simulink model "ofdm_txrx_alamouti"  (Simulink 6.3)
settings: Solver=ode45  StartTime=0.0  StopTime=45000  RelTol=1e-3
InitFcn:
  | ofdm_rx_alamouti_init
StartFcn:
  | ofdm_rx_alamouti_init
values from neighbouring files (symbols this model uses):
  CPLength = 16    [ofdm_tx_alamouti_init.m]
  CRCPolynomial = hex2dec('04c11db7')    [calcTxCRC.m]    (also in ofdm_tx_alamouti_init.m)
  hi = zeros(1,length(cci))    [ofdm_rx_alamouti_init.m]
  hr = zeros(1,length(ccr))    [ofdm_rx_alamouti_init.m]
  max_CP_length = 16    [ofdm_tx_alamouti_init.m]
  max_num_baseRateSymbols = 31    [ofdm_tx_alamouti_init.m]
  max_num_subcarriers = 64    [ofdm_tx_alamouti_init.m]
  max_num_trainingSymbols = 15    [ofdm_tx_alamouti_init.m]
  max_OFDM_symbols = 2047    [ofdm_tx_alamouti_init.m]
  modConstellation_qam16 = 0.75*[1 3 -1 -3]./3    [ofdm_tx_alamouti_init.m]
  modConstellation_qam256 = 0.9375*[3 1 5 7 11 9 13 15 -3 -1 -5 -7 -11 -9 -13 -15]./15    [ofdm_tx_alamouti_init.m]
  modConstellation_qam64 = 0.875*[3 1 5 7 -3 -1 -5 -7]./7    [ofdm_tx_alamouti_init.m]
  modConstellation_qpsk = [1 -1]./sqrt(2)    [ofdm_tx_alamouti_init.m]
  numBaseRateSymbols = 2    [ofdm_tx_alamouti_init.m]
  numFullRateSymbols = numFullRateSymbols + mod(numFullRateSymbols, 2)    [ofdm_tx_alamouti_init.m]
  numSubcarriers = 64    [ofdm_tx_alamouti_init.m]
  numTrainingSymbols = 4    [ofdm_tx_alamouti_init.m]
  pkt_crossCorr_thresh = 0.7    [ofdm_rx_alamouti_init.m]
  pkt_energy_thresh = 0    [ofdm_rx_alamouti_init.m]
  pkt_numPayloadBytes = 24+4+20+20+500    (= 568)    [ofdm_tx_alamouti_init.m]
  pktTiming_count_payloadStart = pktTiming_count_lsStart + pktTiming_ls_num*pktTiming_ls_length    [ofdm_rx_init_packetTimingControl.m]
  preamble = 6*[0 shortsyms_10 longsyms_2]    [ofdm_tx_alamouti_init.m]
  preamble_I = real(preamble)    [ofdm_tx_alamouti_init.m]
  preamble_Q = imag(preamble)    [ofdm_tx_alamouti_init.m]
  RAM_init_size = 4096    [ofdm_tx_alamouti_init.m]
  rx_longCorrCountLoad = 251-16    (= 235)    [ofdm_rx_alamouti_init.m]
  rx_longCorrThresh = 3000    [ofdm_rx_alamouti_init.m]
  RxDataScrambling_Seq = [40 198 78 63 82 173 102 245 48 111 172 115 147 230 216 93 72 65 62 2 205 242 122 90 128 83 105 97 73 10 5 252]    [ofdm_tx_alamouti_init.m]
  simOnly_numSamples = length(preamble)+( (numSubcarriers+CPLength)*(numTrainingSymbols + numBaseRateSymbols + numFullRateSymbols) )    [ofdm_tx_alamouti_init.m]
  train = [train train]    [ofdm_tx_alamouti_init.m]
  TxDataScrambling_Seq = [40 198 78 63 82 173 102 245 48 111 172 115 147 230 216 93 72 65 62 2 205 242 122 90 128 83 105 97 73 10 5 252]    [ofdm_tx_alamouti_init.m]
undefined symbols (not defined in the file or in its folder): CRC_table_gen, gcbh, interpFilter_4, xlBlockLoadCallback
libraries: xbsIndex_r4 x4373, dspmlti4 x4, dspsigops x4, commchan3 x2, simulink x2, commrflib2 x2, commsink2 x2, dspsrcs4 x1
(part 14 of 39)

== system ofdm_txrx_alamouti/OFDM Rx Alamouti/FFT & Chan Est/Channel Estimation/Mult by training ==
  AR : Inport
  AI : Inport  Port=2
  BR : Inport  Port=3
  Constant1 : xbsIndex_r4/Constant  arith_type=Signed (2's comp)  const=0  n_bits=2  bin_pt=1  explicit_period=off  period=1  equ=P=C  opselect=C  inp2=PCIN>>17  opr=+  inp1=P  carry=CIN  dbl_ovrd=off  block_type=constant
      settings: block_version=VER_STRING_GOES_HERE
  Constant2 : xbsIndex_r4/Constant  arith_type=Signed (2's comp)  const=0  n_bits=2  bin_pt=1  explicit_period=off  period=1  equ=P=C  opselect=C  inp2=PCIN>>17  opr=+  inp1=P  carry=CIN  dbl_ovrd=off  block_type=constant
      settings: block_version=VER_STRING_GOES_HERE
  Mult by Training : Scope  NumInputPorts=5
      settings: TimeRange=5000  YMin=-1~-1~-1~0~0  YMax=1~1~1~1~1  SaveName=ScopeData22
  Mux3 : xbsIndex_r4/Mux  inputs=3  en=off  latency=1  precision=Full  arith_type="Signed  (2's comp)"  n_bits=8  bin_pt=2  quantization=Truncate  overflow=Wrap  dbl_ovrd=off  block_type=mux
      settings: xl_area=[0, 0, 0, 0, 0, 0, 0]  block_version=VER_STRING_GOES_HERE
  Mux4 : xbsIndex_r4/Mux  inputs=3  en=off  latency=1  precision=Full  arith_type="Signed  (2's comp)"  n_bits=8  bin_pt=2  quantization=Truncate  overflow=Wrap  dbl_ovrd=off  block_type=mux
      settings: xl_area=[0, 0, 0, 0, 0, 0, 0]  block_version=VER_STRING_GOES_HERE
  Negate : xbsIndex_r4/Negate  precision=User Defined  arith_type="Signed  (2's comp)"  n_bits=16  bin_pt=15  quantization=Truncate  overflow=Flag as error  en=off  latency=0  dbl_ovrd=off  block_type=negate
      settings: xl_area=[0, 0, 0, 0, 0, 0, 0]  block_version=VER_STRING_GOES_HERE
  Negate1 : xbsIndex_r4/Negate  precision=User Defined  arith_type="Signed  (2's comp)"  n_bits=16  bin_pt=15  quantization=Truncate  overflow=Flag as error  en=off  latency=0  dbl_ovrd=off  block_type=negate
      settings: xl_area=[0, 0, 0, 0, 0, 0, 0]  block_version=VER_STRING_GOES_HERE
  Reinterpret : xbsIndex_r4/Reinterpret  force_arith_type=on  arith_type=Unsigned  force_bin_pt=on  bin_pt=0  block_type=cast
      settings: infoedit="Changes type of samples without altering their binary representation.<P><P>Hardware notes: In hardware this block costs nothing.<P><P>Example:  Suppose the input is 6 bits wide, signed, with 2 fractional bits, and the output is forced to unsigned with 0 fractional bits.  Then an input of -2.0 (1110.00 in binary 2's complement) becomes an output of 56 (111000 in binary)."  block_version=VER_STRING_GOES_HERE
  done{1..2,7,12..13} (5 alike) : xbsIndex_r4/Gateway Out  hdl_port=off  timing_constraint=None  locs_specified=off  LOCs={}  block_type=gatewayout
      settings: infoedit="Gateway out block.  Converts Xilinx fixed point inputs into ouputs of type Simulink integer, double, or fixed point.<P><P>Hardware notes:  In hardware these blocks become top level output ports or are discarded, depending on how they are configured."  xl_area=[0 0 0 0 1 0 0]  block_version=VER_STRING_GOES_HERE
  PR : Outport
  PI : Outport  Port=2
  wires:
  AR/1 -> Negate/1, Mux3/3, done12/1
  BR/1 -> Reinterpret/1, done2/1
  AI/1 -> Negate1/1, Mux4/3, done13/1
  Constant1/1 -> Mux4/2
  Negate1/1 -> Mux4/4
  Mux4/1 -> PI/1, done1/1
  Constant2/1 -> Mux3/2
  Negate/1 -> Mux3/4
  Mux3/1 -> PR/1, done7/1
  Reinterpret/1 -> Mux3/1, Mux4/1
  done12/1 -> Mult by Training/2
  done13/1 -> Mult by Training/3
  done2/1 -> Mult by Training/1
  done7/1 -> Mult by Training/4
  done1/1 -> Mult by Training/5

== system ofdm_txrx_alamouti/OFDM Rx Alamouti/FFT & Chan Est/Channel Estimation/WE Gen ==
  Vin : Inport
  Sym Index : Inport  Port=2
  Train : Inport  Port=3
  1LSB : xbsIndex_r4/Slice  nbits=1  boolean_output=on  mode=Lower Bit Location + Width  bit1=0  base1=MSB of Input  bit0=0  base0=LSB of Input  dbl_ovrd=off  block_type=slice
      settings: infoedit="Extracts a given range of bits from each input sample and presents it at the output.  The output type is ordinarily unsigned with binary point at zero, but can be Boolean when the slice is one bit wide.<P><P>Hardware notes: In hardware this block costs nothing."  block_version=VER_STRING_GOES_HERE
  1LSB+1 : xbsIndex_r4/Slice  nbits=1  boolean_output=on  mode=Lower Bit Location + Width  bit1=0  base1=MSB of Input  bit0=1  base0=LSB of Input  dbl_ovrd=off  block_type=slice
      settings: infoedit="Extracts a given range of bits from each input sample and presents it at the output.  The output type is ordinarily unsigned with binary point at zero, but can be Boolean when the slice is one bit wide.<P><P>Hardware notes: In hardware this block costs nothing."  block_version=VER_STRING_GOES_HERE
  Delay{1..4} (4 alike) : xbsIndex_r4/Delay  en=off  latency=1  dbl_ovrd=off  reg_retiming=on  block_type=delay
      settings: infoedit=Hardware notes: A delay line is a chain, each link of which is an SRL16 followed by a flip-flop. If register retiming is enabled, the delay line is a chain of flip-flops.  xl_area=[0, 0, 0, 0, 0, 0, 0]  block_version=VER_STRING_GOES_HERE
  Expression : xbsIndex_r4/Expression  expression=a & b & ~c & ~d  align_bp=on  en=off  latency=0  precision=Full  arith_type=Unsigned  n_bits=16  bin_pt=0  dbl_ovrd=off  block_type=expr
      settings: block_version=VER_STRING_GOES_HERE
  Expression10 : xbsIndex_r4/Expression  expression=a & b & c & d  align_bp=on  en=off  latency=0  precision=Full  arith_type=Unsigned  n_bits=16  bin_pt=0  dbl_ovrd=off  block_type=expr
      settings: block_version=VER_STRING_GOES_HERE
  Expression8 : xbsIndex_r4/Expression  expression=a & b & ~c & d  align_bp=on  en=off  latency=0  precision=Full  arith_type=Unsigned  n_bits=16  bin_pt=0  dbl_ovrd=off  block_type=expr
      settings: block_version=VER_STRING_GOES_HERE
  Expression9 : xbsIndex_r4/Expression  expression=a & b & c & ~d  align_bp=on  en=off  latency=0  precision=Full  arith_type=Unsigned  n_bits=16  bin_pt=0  dbl_ovrd=off  block_type=expr
      settings: block_version=VER_STRING_GOES_HERE
  Logical : xbsIndex_r4/Logical  logical_function=OR  inputs=2  en=off  latency=0  precision=Full  arith_type=Unsigned  n_bits=16  bin_pt=0  align_bp=on  dbl_ovrd=off  block_type=logical
      settings: block_version=8.2.01
  Logical1 : xbsIndex_r4/Logical  logical_function=OR  inputs=2  en=off  latency=0  precision=Full  arith_type=Unsigned  n_bits=16  bin_pt=0  align_bp=on  dbl_ovrd=off  block_type=logical
      settings: block_version=8.2.01
  Seq0 : Outport
  Seq1 : Outport  Port=2
  Seq2 : Outport  Port=3
  Seq3 : Outport  Port=4
  wires:
  Train/1 -> Expression10/2, Expression9/2, Expression8/2, Expression/2
  Sym Index/1 -> 1LSB+1/1, 1LSB/1
  Expression/1 -> Delay3/1
  Expression8/1 -> Delay1/1
  Expression9/1 -> Delay2/1
  Expression10/1 -> Delay4/1
  Vin/1 -> Expression/1, Expression8/1, Expression9/1, Expression10/1
  1LSB/1 -> Expression/4, Expression8/4, Expression9/4, Expression10/4
  Delay3/1 -> Logical/1
  Delay4/1 -> Logical1/2, Seq3/1
  1LSB+1/1 -> Expression10/3, Expression9/3, Expression8/3, Expression/3
  Logical/1 -> Seq0/1
  Delay1/1 -> Logical1/1
  Delay2/1 -> Logical/2, Seq2/1
  Logical1/1 -> Seq1/1

== system ofdm_txrx_alamouti/OFDM Rx Alamouti/FFT & Chan Est/Input Buffers/Antenna A RAM ==
  I : Inport
  Q : Inport  Port=2
  We : Inport  Port=3
  Wr Addr : Inport  Port=4
  Rd Addr : Inport  Port=5
  Constant8 : xbsIndex_r4/Constant  arith_type=Boolean  const=0  n_bits=1  bin_pt=0  explicit_period=off  period=1  equ=P=C  opselect=C  inp2=PCIN>>17  opr=+  inp1=P  carry=CIN  dbl_ovrd=off  block_type=constant
      settings: block_version=VER_STRING_GOES_HERE
  Dual Port RAM : xbsIndex_r4/Dual Port RAM  depth=numSubcarriers + CPLength  initVector=[0.01:0.01:0.80 ones(1,48)]  distributed_mem=Block RAM  init_a=0  init_b=0  rst_a=off  rst_b=off  en_a=off  en_b=off  latency=1  write_mode_A=Read After Write  write_mode_B=Read After Write  dbl_ovrd=off  optimize=Area  use_rpm=off  block_type=dpram
      settings: xl_area=[0 0 1 0 0 0 0]  block_version=VER_STRING_GOES_HERE
  Dual Port RAM1 : xbsIndex_r4/Dual Port RAM  depth=numSubcarriers + CPLength  initVector=[0.80:-0.01:0.01 ones(1,48)]  distributed_mem=Block RAM  init_a=0  init_b=0  rst_a=off  rst_b=off  en_a=off  en_b=off  latency=1  write_mode_A=Read After Write  write_mode_B=Read After Write  dbl_ovrd=off  optimize=Area  use_rpm=off  block_type=dpram
      settings: xl_area=[0 0 1 0 0 0 0]  block_version=VER_STRING_GOES_HERE
  " I" : Outport
  " Q" : Outport  Port=2
  wires:
  We/1 -> Dual Port RAM/3, Dual Port RAM1/3
  Q/1 -> Dual Port RAM1/2, Dual Port RAM1/5
  I/1 -> Dual Port RAM/2, Dual Port RAM/5
  Dual Port RAM/2 -> " I"/1
  Dual Port RAM1/2 -> " Q"/1
  Rd Addr/1 -> Dual Port RAM/4, Dual Port RAM1/4
  Constant8/1 -> Dual Port RAM1/6, Dual Port RAM/6
  Wr Addr/1 -> Dual Port RAM/1, Dual Port RAM1/1

== system ofdm_txrx_alamouti/OFDM Rx Alamouti/FFT & Chan Est/Input Buffers/Antenna B RAM ==
  I : Inport
  Q : Inport  Port=2
  We : Inport  Port=3
  Wr Addr : Inport  Port=4
  Rd Addr : Inport  Port=5
  Constant8 : xbsIndex_r4/Constant  arith_type=Boolean  const=0  n_bits=1  bin_pt=0  explicit_period=off  period=1  equ=P=C  opselect=C  inp2=PCIN>>17  opr=+  inp1=P  carry=CIN  dbl_ovrd=off  block_type=constant
      settings: block_version=VER_STRING_GOES_HERE
  Dual Port RAM : xbsIndex_r4/Dual Port RAM  depth=numSubcarriers + CPLength  initVector=[0.01:0.01:0.80 ones(1,48)]  distributed_mem=Block RAM  init_a=0  init_b=0  rst_a=off  rst_b=off  en_a=off  en_b=off  latency=1  write_mode_A=Read After Write  write_mode_B=Read After Write  dbl_ovrd=off  optimize=Area  use_rpm=off  block_type=dpram
      settings: xl_area=[0 0 1 0 0 0 0]  block_version=VER_STRING_GOES_HERE
  Dual Port RAM1 : xbsIndex_r4/Dual Port RAM  depth=numSubcarriers + CPLength  initVector=[0.80:-0.01:0.01 ones(1,48)]  distributed_mem=Block RAM  init_a=0  init_b=0  rst_a=off  rst_b=off  en_a=off  en_b=off  latency=1  write_mode_A=Read After Write  write_mode_B=Read After Write  dbl_ovrd=off  optimize=Area  use_rpm=off  block_type=dpram
      settings: xl_area=[0 0 1 0 0 0 0]  block_version=VER_STRING_GOES_HERE
  " I" : Outport
  " Q" : Outport  Port=2
  wires:
  Wr Addr/1 -> Dual Port RAM1/1, Dual Port RAM/1
  Constant8/1 -> Dual Port RAM/6, Dual Port RAM1/6
  Rd Addr/1 -> Dual Port RAM1/4, Dual Port RAM/4
  Dual Port RAM1/2 -> " Q"/1
  Dual Port RAM/2 -> " I"/1
  I/1 -> Dual Port RAM/5, Dual Port RAM/2
  Q/1 -> Dual Port RAM1/5, Dual Port RAM1/2
  We/1 -> Dual Port RAM1/3, Dual Port RAM/3

== system ofdm_txrx_alamouti/OFDM Rx Alamouti/FFT & Chan Est/Output Buffers/AntA Buffer ==
  I : Inport
  Q : Inport  Port=2
  We : Inport  Port=3
  Wr Addr : Inport  Port=4
  Rd Addr : Inport  Port=5
  Constant8 : xbsIndex_r4/Constant  arith_type=Boolean  const=0  n_bits=1  bin_pt=0  explicit_period=off  period=1  equ=P=C  opselect=C  inp2=PCIN>>17  opr=+  inp1=P  carry=CIN  dbl_ovrd=off  block_type=constant
      settings: block_version=VER_STRING_GOES_HERE
  Dual Port RAM : xbsIndex_r4/Dual Port RAM  depth=numSubcarriers  initVector=[0.01:0.01:0.80 ones(1,48)]  distributed_mem=Block RAM  init_a=0  init_b=0  rst_a=off  rst_b=off  en_a=off  en_b=off  latency=1  write_mode_A=Read After Write  write_mode_B=Read After Write  dbl_ovrd=off  optimize=Area  use_rpm=off  block_type=dpram
      settings: xl_area=[0 0 1 0 0 0 0]  block_version=VER_STRING_GOES_HERE
  Dual Port RAM1 : xbsIndex_r4/Dual Port RAM  depth=numSubcarriers  initVector=[0.80:-0.01:0.01 ones(1,48)]  distributed_mem=Block RAM  init_a=0  init_b=0  rst_a=off  rst_b=off  en_a=off  en_b=off  latency=1  write_mode_A=Read After Write  write_mode_B=Read After Write  dbl_ovrd=off  optimize=Area  use_rpm=off  block_type=dpram
      settings: xl_area=[0 0 1 0 0 0 0]  block_version=VER_STRING_GOES_HERE
  " I" : Outport
  " Q" : Outport  Port=2
  wires:
  Wr Addr/1 -> Dual Port RAM1/1, Dual Port RAM/1
  Constant8/1 -> Dual Port RAM/6, Dual Port RAM1/6
  Rd Addr/1 -> Dual Port RAM1/4, Dual Port RAM/4
  Dual Port RAM1/2 -> " Q"/1
  Dual Port RAM/2 -> " I"/1
  I/1 -> Dual Port RAM/5, Dual Port RAM/2
  Q/1 -> Dual Port RAM1/5, Dual Port RAM1/2
  We/1 -> Dual Port RAM1/3, Dual Port RAM/3

== system ofdm_txrx_alamouti/OFDM Rx Alamouti/FFT & Chan Est/Output Buffers/AntB Buffer ==
  I : Inport
  Q : Inport  Port=2
  We : Inport  Port=3
  Wr Addr : Inport  Port=4
  Rd Addr : Inport  Port=5
  Constant8 : xbsIndex_r4/Constant  arith_type=Boolean  const=0  n_bits=1  bin_pt=0  explicit_period=off  period=1  equ=P=C  opselect=C  inp2=PCIN>>17  opr=+  inp1=P  carry=CIN  dbl_ovrd=off  block_type=constant
      settings: block_version=VER_STRING_GOES_HERE
  Dual Port RAM : xbsIndex_r4/Dual Port RAM  depth=numSubcarriers  initVector=[0.01:0.01:0.80 ones(1,48)]  distributed_mem=Block RAM  init_a=0  init_b=0  rst_a=off  rst_b=off  en_a=off  en_b=off  latency=1  write_mode_A=Read After Write  write_mode_B=Read After Write  dbl_ovrd=off  optimize=Area  use_rpm=off  block_type=dpram
      settings: xl_area=[0 0 1 0 0 0 0]  block_version=VER_STRING_GOES_HERE
  Dual Port RAM1 : xbsIndex_r4/Dual Port RAM  depth=numSubcarriers  initVector=[0.80:-0.01:0.01 ones(1,48)]  distributed_mem=Block RAM  init_a=0  init_b=0  rst_a=off  rst_b=off  en_a=off  en_b=off  latency=1  write_mode_A=Read After Write  write_mode_B=Read After Write  dbl_ovrd=off  optimize=Area  use_rpm=off  block_type=dpram
      settings: xl_area=[0 0 1 0 0 0 0]  block_version=VER_STRING_GOES_HERE
  " I" : Outport
  " Q" : Outport  Port=2
  wires:
  We/1 -> Dual Port RAM/3, Dual Port RAM1/3
  Q/1 -> Dual Port RAM1/2, Dual Port RAM1/5
  I/1 -> Dual Port RAM/2, Dual Port RAM/5
  Dual Port RAM/2 -> " I"/1
  Dual Port RAM1/2 -> " Q"/1
  Rd Addr/1 -> Dual Port RAM/4, Dual Port RAM1/4
  Constant8/1 -> Dual Port RAM1/6, Dual Port RAM/6
  Wr Addr/1 -> Dual Port RAM/1, Dual Port RAM1/1

== system ofdm_txrx_alamouti/OFDM Rx Alamouti/FFT & Chan Est/Symbol Counter/Posedge Det ==
  D : Inport
  Delay : xbsIndex_r4/Delay  en=off  latency=1  dbl_ovrd=off  reg_retiming=off  block_type=delay
      settings: infoedit=Hardware notes: A delay line is a chain, each link of which is an SRL16 followed by a flip-flop. If register retiming is enabled, the delay line is a chain of flip-flops.  xl_area=[1 1 0 0 0 0 0]  block_version=VER_STRING_GOES_HERE
  Inverter : xbsIndex_r4/Inverter  en=off  latency=0  dbl_ovrd=off  block_type=inv
      settings: xl_area=[0 0 0 0 0 0 0]  block_version=VER_STRING_GOES_HERE
  Logical : xbsIndex_r4/Logical  logical_function=AND  inputs=2  en=off  latency=0  precision=Full  arith_type=Unsigned  n_bits=8  bin_pt=2  align_bp=on  dbl_ovrd=off  block_type=logical
      settings: xl_area=[1 0 0 1 0 0 0]  block_version=VER_STRING_GOES_HERE
  Q : Outport
  wires:
  Delay/1 -> Inverter/1
  Inverter/1 -> Logical/2
  D/1 -> Logical/1, Delay/1
  Logical/1 -> Q/1

== system ofdm_txrx_alamouti/OFDM Rx Alamouti/FFT & Chan Est/Symbol Counter/S-R Latch ==
  S : Inport
  R : Inport  Port=2
  Constant2 : xbsIndex_r4/Constant  arith_type=Boolean  const=1  n_bits=1  bin_pt=0  explicit_period=off  period=1  equ=P=C  opselect=C  inp2=PCIN>>17  opr=+  inp1=P  carry=CIN  dbl_ovrd=off  block_type=constant
      settings: block_version=VER_STRING_GOES_HERE
  Register : xbsIndex_r4/Register  init=0  rst=on  en=on  dbl_ovrd=off  block_type=register
      settings: xl_area=[0, 0, 0, 0, 0, 0, 0]  block_version=VER_STRING_GOES_HERE
  D : Outport
  wires:
  Constant2/1 -> Register/1
  R/1 -> Register/2
  S/1 -> Register/3
  Register/1 -> D/1

== system ofdm_txrx_alamouti/OFDM Rx Alamouti/Long Corr 4x/C1/Cross C1 ==
  xn : Inport
  DAddr : Inport  Port=2
  CAddr : Inport  Port=3
  LD : Inport  Port=4
  Down Sample1 : xbsIndex_r4/Down Sample  sample_ratio=4  sample_phase=First Value of Frame  en=off  latency=0  dbl_ovrd=off  block_type=dsamp
      settings: xl_area=[5 5 0 4 0 0 0]  block_version=VER_STRING_GOES_HERE
  Down Sample2 : xbsIndex_r4/Down Sample  sample_ratio=length(coef2)  sample_phase=First Value of Frame  en=off  latency=0  dbl_ovrd=off  block_type=dsamp
      settings: xl_area=[5 5 0 4 0 0 0]  block_version=VER_STRING_GOES_HERE
  MAC : Subsystem
  R : xbsIndex_r4/Addressable Shift Register  infer_latency=off  depth=length(coef2)  initVector=[0]  en=off  dbl_ovrd=off  use_behavioral_HDL=off  use_rpm=off  block_type=addrsr
      settings: infoedit="Delay of  configurable length.  Any element  in the delay     line   can    be   addressed   and   driven    onto   the   output     port.<P><P>Hardware  notes: Implemented  using SRL16s.     If Virtex-4, Virtex-II or Spartan-3 devices are used, multiple SRLC16s are cascaded together."  xl_area=[1 0 0 2 0 0 0]  block_version=VER_STRING_GOES_HERE
  ROM1 : xbsIndex_r4/ROM  depth=length(coef2)  initVector=coef2  distributed_mem=Distributed memory  rst=off  init_reg=0  en=off  latency=1  arith_type=Unsigned  n_bits=1  bin_pt=0  dbl_ovrd=off  optimize=Area  use_rpm=off  block_type=sprom
      settings: xl_area=[1 1 0 1 0 0 0]  block_version=VER_STRING_GOES_HERE
  yn : Outport
  xnz : Outport  Port=2
  wires:
  Down Sample1/1 -> R/1
  LD/1 -> MAC/3(ld)
  ROM1/1 -> MAC/2(coeff)
  Down Sample2/1 -> yn/1
  MAC/1(yn) -> Down Sample2/1
  R/1 -> MAC/1(a), xnz/1
  CAddr/1 -> ROM1/1
  DAddr/1 -> R/2
  xn/1 -> Down Sample1/1

== system ofdm_txrx_alamouti/OFDM Rx Alamouti/Long Corr 4x/C1/Cross C10 ==
  xn : Inport
  DAddr : Inport  Port=2
  CAddr : Inport  Port=3
  LD : Inport  Port=4
  Down Sample1 : xbsIndex_r4/Down Sample  sample_ratio=4  sample_phase=First Value of Frame  en=off  latency=0  dbl_ovrd=off  block_type=dsamp
      settings: xl_area=[5 5 0 4 0 0 0]  block_version=VER_STRING_GOES_HERE
  Down Sample2 : xbsIndex_r4/Down Sample  sample_ratio=length(coef2)  sample_phase=First Value of Frame  en=off  latency=0  dbl_ovrd=off  block_type=dsamp
      settings: xl_area=[5 5 0 4 0 0 0]  block_version=VER_STRING_GOES_HERE
  MAC : Subsystem
  R : xbsIndex_r4/Addressable Shift Register  infer_latency=off  depth=length(coef2)  initVector=[0]  en=off  dbl_ovrd=off  use_behavioral_HDL=off  use_rpm=off  block_type=addrsr
      settings: infoedit="Delay of  configurable length.  Any element  in the delay     line   can    be   addressed   and   driven    onto   the   output     port.<P><P>Hardware  notes: Implemented  using SRL16s.     If Virtex-4, Virtex-II or Spartan-3 devices are used, multiple SRLC16s are cascaded together."  xl_area=[1 0 0 2 0 0 0]  block_version=VER_STRING_GOES_HERE
  ROM1 : xbsIndex_r4/ROM  depth=length(coef2)  initVector=coef2  distributed_mem=Distributed memory  rst=off  init_reg=0  en=off  latency=1  arith_type=Unsigned  n_bits=1  bin_pt=0  dbl_ovrd=off  optimize=Area  use_rpm=off  block_type=sprom
      settings: xl_area=[1 1 0 1 0 0 0]  block_version=VER_STRING_GOES_HERE
  yn : Outport
  xnz : Outport  Port=2
  wires:
  Down Sample1/1 -> R/1
  LD/1 -> MAC/3(ld)
  ROM1/1 -> MAC/2(coeff)
  Down Sample2/1 -> yn/1
  MAC/1(yn) -> Down Sample2/1
  R/1 -> MAC/1(a), xnz/1
  CAddr/1 -> ROM1/1
  DAddr/1 -> R/2
  xn/1 -> Down Sample1/1

== system ofdm_txrx_alamouti/OFDM Rx Alamouti/Long Corr 4x/C1/Cross C11 ==
  xn : Inport
  DAddr : Inport  Port=2
  CAddr : Inport  Port=3
  LD : Inport  Port=4
  Down Sample1 : xbsIndex_r4/Down Sample  sample_ratio=4  sample_phase=First Value of Frame  en=off  latency=0  dbl_ovrd=off  block_type=dsamp
      settings: xl_area=[5 5 0 4 0 0 0]  block_version=VER_STRING_GOES_HERE
  Down Sample2 : xbsIndex_r4/Down Sample  sample_ratio=length(coef2)  sample_phase=First Value of Frame  en=off  latency=0  dbl_ovrd=off  block_type=dsamp
      settings: xl_area=[5 5 0 4 0 0 0]  block_version=VER_STRING_GOES_HERE
  MAC : Subsystem
  R : xbsIndex_r4/Addressable Shift Register  infer_latency=off  depth=length(coef2)  initVector=[0]  en=off  dbl_ovrd=off  use_behavioral_HDL=off  use_rpm=off  block_type=addrsr
      settings: infoedit="Delay of  configurable length.  Any element  in the delay     line   can    be   addressed   and   driven    onto   the   output     port.<P><P>Hardware  notes: Implemented  using SRL16s.     If Virtex-4, Virtex-II or Spartan-3 devices are used, multiple SRLC16s are cascaded together."  xl_area=[1 0 0 2 0 0 0]  block_version=VER_STRING_GOES_HERE
  ROM1 : xbsIndex_r4/ROM  depth=length(coef2)  initVector=coef2  distributed_mem=Distributed memory  rst=off  init_reg=0  en=off  latency=1  arith_type=Unsigned  n_bits=1  bin_pt=0  dbl_ovrd=off  optimize=Area  use_rpm=off  block_type=sprom
      settings: xl_area=[1 1 0 1 0 0 0]  block_version=VER_STRING_GOES_HERE
  yn : Outport
  xnz : Outport  Port=2
  wires:
  Down Sample1/1 -> R/1
  LD/1 -> MAC/3(ld)
  ROM1/1 -> MAC/2(coeff)
  Down Sample2/1 -> yn/1
  MAC/1(yn) -> Down Sample2/1
  R/1 -> MAC/1(a), xnz/1
  CAddr/1 -> ROM1/1
  DAddr/1 -> R/2
  xn/1 -> Down Sample1/1

== system ofdm_txrx_alamouti/OFDM Rx Alamouti/Long Corr 4x/C1/Cross C12 ==
  xn : Inport
  DAddr : Inport  Port=2
  CAddr : Inport  Port=3
  LD : Inport  Port=4
  Down Sample1 : xbsIndex_r4/Down Sample  sample_ratio=4  sample_phase=First Value of Frame  en=off  latency=0  dbl_ovrd=off  block_type=dsamp
      settings: xl_area=[5 5 0 4 0 0 0]  block_version=VER_STRING_GOES_HERE
  Down Sample2 : xbsIndex_r4/Down Sample  sample_ratio=length(coef2)  sample_phase=First Value of Frame  en=off  latency=0  dbl_ovrd=off  block_type=dsamp
      settings: xl_area=[5 5 0 4 0 0 0]  block_version=VER_STRING_GOES_HERE
  MAC : Subsystem
  R : xbsIndex_r4/Addressable Shift Register  infer_latency=off  depth=length(coef2)  initVector=[0]  en=off  dbl_ovrd=off  use_behavioral_HDL=off  use_rpm=off  block_type=addrsr
      settings: infoedit="Delay of  configurable length.  Any element  in the delay     line   can    be   addressed   and   driven    onto   the   output     port.<P><P>Hardware  notes: Implemented  using SRL16s.     If Virtex-4, Virtex-II or Spartan-3 devices are used, multiple SRLC16s are cascaded together."  xl_area=[1 0 0 2 0 0 0]  block_version=VER_STRING_GOES_HERE
  ROM1 : xbsIndex_r4/ROM  depth=length(coef2)  initVector=coef2  distributed_mem=Distributed memory  rst=off  init_reg=0  en=off  latency=1  arith_type=Unsigned  n_bits=1  bin_pt=0  dbl_ovrd=off  optimize=Area  use_rpm=off  block_type=sprom
      settings: xl_area=[1 1 0 1 0 0 0]  block_version=VER_STRING_GOES_HERE
  yn : Outport
  xnz : Outport  Port=2
  wires:
  Down Sample1/1 -> R/1
  LD/1 -> MAC/3(ld)
  ROM1/1 -> MAC/2(coeff)
  Down Sample2/1 -> yn/1
  MAC/1(yn) -> Down Sample2/1
  R/1 -> MAC/1(a), xnz/1
  CAddr/1 -> ROM1/1
  DAddr/1 -> R/2
  xn/1 -> Down Sample1/1

== system ofdm_txrx_alamouti/OFDM Rx Alamouti/Long Corr 4x/C1/Cross C13 ==
  xn : Inport
  DAddr : Inport  Port=2
  CAddr : Inport  Port=3
  LD : Inport  Port=4
  Down Sample1 : xbsIndex_r4/Down Sample  sample_ratio=4  sample_phase=First Value of Frame  en=off  latency=0  dbl_ovrd=off  block_type=dsamp
      settings: xl_area=[5 5 0 4 0 0 0]  block_version=VER_STRING_GOES_HERE
  Down Sample2 : xbsIndex_r4/Down Sample  sample_ratio=length(coef2)  sample_phase=First Value of Frame  en=off  latency=0  dbl_ovrd=off  block_type=dsamp
      settings: xl_area=[5 5 0 4 0 0 0]  block_version=VER_STRING_GOES_HERE
  MAC : Subsystem
  R : xbsIndex_r4/Addressable Shift Register  infer_latency=off  depth=length(coef2)  initVector=[0]  en=off  dbl_ovrd=off  use_behavioral_HDL=off  use_rpm=off  block_type=addrsr
      settings: infoedit="Delay of  configurable length.  Any element  in the delay     line   can    be   addressed   and   driven    onto   the   output     port.<P><P>Hardware  notes: Implemented  using SRL16s.     If Virtex-4, Virtex-II or Spartan-3 devices are used, multiple SRLC16s are cascaded together."  xl_area=[1 0 0 2 0 0 0]  block_version=VER_STRING_GOES_HERE
  ROM1 : xbsIndex_r4/ROM  depth=length(coef2)  initVector=coef2  distributed_mem=Distributed memory  rst=off  init_reg=0  en=off  latency=1  arith_type=Unsigned  n_bits=1  bin_pt=0  dbl_ovrd=off  optimize=Area  use_rpm=off  block_type=sprom
      settings: xl_area=[1 1 0 1 0 0 0]  block_version=VER_STRING_GOES_HERE
  yn : Outport
  xnz : Outport  Port=2
  wires:
  Down Sample1/1 -> R/1
  LD/1 -> MAC/3(ld)
  ROM1/1 -> MAC/2(coeff)
  Down Sample2/1 -> yn/1
  MAC/1(yn) -> Down Sample2/1
  R/1 -> MAC/1(a), xnz/1
  CAddr/1 -> ROM1/1
  DAddr/1 -> R/2
  xn/1 -> Down Sample1/1

== system ofdm_txrx_alamouti/OFDM Rx Alamouti/Long Corr 4x/C1/Cross C14 ==
  xn : Inport
  DAddr : Inport  Port=2
  CAddr : Inport  Port=3
  LD : Inport  Port=4
  Down Sample1 : xbsIndex_r4/Down Sample  sample_ratio=4  sample_phase=First Value of Frame  en=off  latency=0  dbl_ovrd=off  block_type=dsamp
      settings: xl_area=[5 5 0 4 0 0 0]  block_version=VER_STRING_GOES_HERE
  Down Sample2 : xbsIndex_r4/Down Sample  sample_ratio=length(coef2)  sample_phase=First Value of Frame  en=off  latency=0  dbl_ovrd=off  block_type=dsamp
      settings: xl_area=[5 5 0 4 0 0 0]  block_version=VER_STRING_GOES_HERE
  MAC : Subsystem
  R : xbsIndex_r4/Addressable Shift Register  infer_latency=off  depth=length(coef2)  initVector=[0]  en=off  dbl_ovrd=off  use_behavioral_HDL=off  use_rpm=off  block_type=addrsr
      settings: infoedit="Delay of  configurable length.  Any element  in the delay     line   can    be   addressed   and   driven    onto   the   output     port.<P><P>Hardware  notes: Implemented  using SRL16s.     If Virtex-4, Virtex-II or Spartan-3 devices are used, multiple SRLC16s are cascaded together."  xl_area=[1 0 0 2 0 0 0]  block_version=VER_STRING_GOES_HERE
  ROM1 : xbsIndex_r4/ROM  depth=length(coef2)  initVector=coef2  distributed_mem=Distributed memory  rst=off  init_reg=0  en=off  latency=1  arith_type=Unsigned  n_bits=1  bin_pt=0  dbl_ovrd=off  optimize=Area  use_rpm=off  block_type=sprom
      settings: xl_area=[1 1 0 1 0 0 0]  block_version=VER_STRING_GOES_HERE
  yn : Outport
  xnz : Outport  Port=2
  wires:
  Down Sample1/1 -> R/1
  LD/1 -> MAC/3(ld)
  ROM1/1 -> MAC/2(coeff)
  Down Sample2/1 -> yn/1
  MAC/1(yn) -> Down Sample2/1
  R/1 -> MAC/1(a), xnz/1
  CAddr/1 -> ROM1/1
  DAddr/1 -> R/2
  xn/1 -> Down Sample1/1

== system ofdm_txrx_alamouti/OFDM Rx Alamouti/Long Corr 4x/C1/Cross C15 ==
  xn : Inport
  DAddr : Inport  Port=2
  CAddr : Inport  Port=3
  LD : Inport  Port=4
  Down Sample1 : xbsIndex_r4/Down Sample  sample_ratio=4  sample_phase=First Value of Frame  en=off  latency=0  dbl_ovrd=off  block_type=dsamp
      settings: xl_area=[5 5 0 4 0 0 0]  block_version=VER_STRING_GOES_HERE
  Down Sample2 : xbsIndex_r4/Down Sample  sample_ratio=length(coef2)  sample_phase=First Value of Frame  en=off  latency=0  dbl_ovrd=off  block_type=dsamp
      settings: xl_area=[5 5 0 4 0 0 0]  block_version=VER_STRING_GOES_HERE
  MAC : Subsystem
  R : xbsIndex_r4/Addressable Shift Register  infer_latency=off  depth=length(coef2)  initVector=[0]  en=off  dbl_ovrd=off  use_behavioral_HDL=off  use_rpm=off  block_type=addrsr
      settings: infoedit="Delay of  configurable length.  Any element  in the delay     line   can    be   addressed   and   driven    onto   the   output     port.<P><P>Hardware  notes: Implemented  using SRL16s.     If Virtex-4, Virtex-II or Spartan-3 devices are used, multiple SRLC16s are cascaded together."  xl_area=[1 0 0 2 0 0 0]  block_version=VER_STRING_GOES_HERE
  ROM1 : xbsIndex_r4/ROM  depth=length(coef2)  initVector=coef2  distributed_mem=Distributed memory  rst=off  init_reg=0  en=off  latency=1  arith_type=Unsigned  n_bits=1  bin_pt=0  dbl_ovrd=off  optimize=Area  use_rpm=off  block_type=sprom
      settings: xl_area=[1 1 0 1 0 0 0]  block_version=VER_STRING_GOES_HERE
  yn : Outport
  xnz : Outport  Port=2
  wires:
  Down Sample1/1 -> R/1
  LD/1 -> MAC/3(ld)
  ROM1/1 -> MAC/2(coeff)
  Down Sample2/1 -> yn/1
  MAC/1(yn) -> Down Sample2/1
  R/1 -> MAC/1(a), xnz/1
  CAddr/1 -> ROM1/1
  DAddr/1 -> R/2
  xn/1 -> Down Sample1/1

== system ofdm_txrx_alamouti/OFDM Rx Alamouti/Long Corr 4x/C1/Cross C16 ==
  xn : Inport
  DAddr : Inport  Port=2
  CAddr : Inport  Port=3
  LD : Inport  Port=4
  Down Sample1 : xbsIndex_r4/Down Sample  sample_ratio=4  sample_phase=First Value of Frame  en=off  latency=0  dbl_ovrd=off  block_type=dsamp
      settings: xl_area=[5 5 0 4 0 0 0]  block_version=VER_STRING_GOES_HERE
  Down Sample2 : xbsIndex_r4/Down Sample  sample_ratio=length(coef2)  sample_phase=First Value of Frame  en=off  latency=0  dbl_ovrd=off  block_type=dsamp
      settings: xl_area=[5 5 0 4 0 0 0]  block_version=VER_STRING_GOES_HERE
  MAC : Subsystem
  R : xbsIndex_r4/Addressable Shift Register  infer_latency=off  depth=length(coef2)  initVector=[0]  en=off  dbl_ovrd=off  use_behavioral_HDL=off  use_rpm=off  block_type=addrsr
      settings: infoedit="Delay of  configurable length.  Any element  in the delay     line   can    be   addressed   and   driven    onto   the   output     port.<P><P>Hardware  notes: Implemented  using SRL16s.     If Virtex-4, Virtex-II or Spartan-3 devices are used, multiple SRLC16s are cascaded together."  xl_area=[1 0 0 2 0 0 0]  block_version=VER_STRING_GOES_HERE
  ROM1 : xbsIndex_r4/ROM  depth=length(coef2)  initVector=coef2  distributed_mem=Distributed memory  rst=off  init_reg=0  en=off  latency=1  arith_type=Unsigned  n_bits=1  bin_pt=0  dbl_ovrd=off  optimize=Area  use_rpm=off  block_type=sprom
      settings: xl_area=[1 1 0 1 0 0 0]  block_version=VER_STRING_GOES_HERE
  yn : Outport
  xnz : Outport  Port=2
  wires:
  Down Sample1/1 -> R/1
  LD/1 -> MAC/3(ld)
  ROM1/1 -> MAC/2(coeff)
  Down Sample2/1 -> yn/1
  MAC/1(yn) -> Down Sample2/1
  R/1 -> MAC/1(a), xnz/1
  CAddr/1 -> ROM1/1
  DAddr/1 -> R/2
  xn/1 -> Down Sample1/1

== system ofdm_txrx_alamouti/OFDM Rx Alamouti/Long Corr 4x/C1/Cross C2 ==
  xn : Inport
  DAddr : Inport  Port=2
  CAddr : Inport  Port=3
  LD : Inport  Port=4
  Down Sample1 : xbsIndex_r4/Down Sample  sample_ratio=4  sample_phase=First Value of Frame  en=off  latency=0  dbl_ovrd=off  block_type=dsamp
      settings: xl_area=[5 5 0 4 0 0 0]  block_version=VER_STRING_GOES_HERE
  Down Sample2 : xbsIndex_r4/Down Sample  sample_ratio=length(coef2)  sample_phase=First Value of Frame  en=off  latency=0  dbl_ovrd=off  block_type=dsamp
      settings: xl_area=[5 5 0 4 0 0 0]  block_version=VER_STRING_GOES_HERE
  MAC : Subsystem
  R : xbsIndex_r4/Addressable Shift Register  infer_latency=off  depth=length(coef2)  initVector=[0]  en=off  dbl_ovrd=off  use_behavioral_HDL=off  use_rpm=off  block_type=addrsr
      settings: infoedit="Delay of  configurable length.  Any element  in the delay     line   can    be   addressed   and   driven    onto   the   output     port.<P><P>Hardware  notes: Implemented  using SRL16s.     If Virtex-4, Virtex-II or Spartan-3 devices are used, multiple SRLC16s are cascaded together."  xl_area=[1 0 0 2 0 0 0]  block_version=VER_STRING_GOES_HERE
  ROM1 : xbsIndex_r4/ROM  depth=length(coef2)  initVector=coef2  distributed_mem=Distributed memory  rst=off  init_reg=0  en=off  latency=1  arith_type=Unsigned  n_bits=1  bin_pt=0  dbl_ovrd=off  optimize=Area  use_rpm=off  block_type=sprom
      settings: xl_area=[1 1 0 1 0 0 0]  block_version=VER_STRING_GOES_HERE
  yn : Outport
  xnz : Outport  Port=2
  wires:
  Down Sample1/1 -> R/1
  LD/1 -> MAC/3(ld)
  ROM1/1 -> MAC/2(coeff)
  Down Sample2/1 -> yn/1
  MAC/1(yn) -> Down Sample2/1
  R/1 -> MAC/1(a), xnz/1
  CAddr/1 -> ROM1/1
  DAddr/1 -> R/2
  xn/1 -> Down Sample1/1

== system ofdm_txrx_alamouti/OFDM Rx Alamouti/Long Corr 4x/C1/Cross C3 ==
  xn : Inport
  DAddr : Inport  Port=2
  CAddr : Inport  Port=3
  LD : Inport  Port=4
  Down Sample1 : xbsIndex_r4/Down Sample  sample_ratio=4  sample_phase=First Value of Frame  en=off  latency=0  dbl_ovrd=off  block_type=dsamp
      settings: xl_area=[5 5 0 4 0 0 0]  block_version=VER_STRING_GOES_HERE
  Down Sample2 : xbsIndex_r4/Down Sample  sample_ratio=length(coef2)  sample_phase=First Value of Frame  en=off  latency=0  dbl_ovrd=off  block_type=dsamp
      settings: xl_area=[5 5 0 4 0 0 0]  block_version=VER_STRING_GOES_HERE
  MAC : Subsystem
  R : xbsIndex_r4/Addressable Shift Register  infer_latency=off  depth=length(coef2)  initVector=[0]  en=off  dbl_ovrd=off  use_behavioral_HDL=off  use_rpm=off  block_type=addrsr
      settings: infoedit="Delay of  configurable length.  Any element  in the delay     line   can    be   addressed   and   driven    onto   the   output     port.<P><P>Hardware  notes: Implemented  using SRL16s.     If Virtex-4, Virtex-II or Spartan-3 devices are used, multiple SRLC16s are cascaded together."  xl_area=[1 0 0 2 0 0 0]  block_version=VER_STRING_GOES_HERE
  ROM1 : xbsIndex_r4/ROM  depth=length(coef2)  initVector=coef2  distributed_mem=Distributed memory  rst=off  init_reg=0  en=off  latency=1  arith_type=Unsigned  n_bits=1  bin_pt=0  dbl_ovrd=off  optimize=Area  use_rpm=off  block_type=sprom
      settings: xl_area=[1 1 0 1 0 0 0]  block_version=VER_STRING_GOES_HERE
  yn : Outport
  xnz : Outport  Port=2
  wires:
  Down Sample1/1 -> R/1
  LD/1 -> MAC/3(ld)
  ROM1/1 -> MAC/2(coeff)
  Down Sample2/1 -> yn/1
  MAC/1(yn) -> Down Sample2/1
  R/1 -> MAC/1(a), xnz/1
  CAddr/1 -> ROM1/1
  DAddr/1 -> R/2
  xn/1 -> Down Sample1/1

== system ofdm_txrx_alamouti/OFDM Rx Alamouti/Long Corr 4x/C1/Cross C4 ==
  xn : Inport
  DAddr : Inport  Port=2
  CAddr : Inport  Port=3
  LD : Inport  Port=4
  Down Sample1 : xbsIndex_r4/Down Sample  sample_ratio=4  sample_phase=First Value of Frame  en=off  latency=0  dbl_ovrd=off  block_type=dsamp
      settings: xl_area=[5 5 0 4 0 0 0]  block_version=VER_STRING_GOES_HERE
  Down Sample2 : xbsIndex_r4/Down Sample  sample_ratio=length(coef2)  sample_phase=First Value of Frame  en=off  latency=0  dbl_ovrd=off  block_type=dsamp
      settings: xl_area=[5 5 0 4 0 0 0]  block_version=VER_STRING_GOES_HERE
  MAC : Subsystem
  R : xbsIndex_r4/Addressable Shift Register  infer_latency=off  depth=length(coef2)  initVector=[0]  en=off  dbl_ovrd=off  use_behavioral_HDL=off  use_rpm=off  block_type=addrsr
      settings: infoedit="Delay of  configurable length.  Any element  in the delay     line   can    be   addressed   and   driven    onto   the   output     port.<P><P>Hardware  notes: Implemented  using SRL16s.     If Virtex-4, Virtex-II or Spartan-3 devices are used, multiple SRLC16s are cascaded together."  xl_area=[1 0 0 2 0 0 0]  block_version=VER_STRING_GOES_HERE
  ROM1 : xbsIndex_r4/ROM  depth=length(coef2)  initVector=coef2  distributed_mem=Distributed memory  rst=off  init_reg=0  en=off  latency=1  arith_type=Unsigned  n_bits=1  bin_pt=0  dbl_ovrd=off  optimize=Area  use_rpm=off  block_type=sprom
      settings: xl_area=[1 1 0 1 0 0 0]  block_version=VER_STRING_GOES_HERE
  yn : Outport
  xnz : Outport  Port=2
  wires:
  Down Sample1/1 -> R/1
  LD/1 -> MAC/3(ld)
  ROM1/1 -> MAC/2(coeff)
  Down Sample2/1 -> yn/1
  MAC/1(yn) -> Down Sample2/1
  R/1 -> MAC/1(a), xnz/1
  CAddr/1 -> ROM1/1
  DAddr/1 -> R/2
  xn/1 -> Down Sample1/1

== system ofdm_txrx_alamouti/OFDM Rx Alamouti/Long Corr 4x/C1/Cross C5 ==
  xn : Inport
  DAddr : Inport  Port=2
  CAddr : Inport  Port=3
  LD : Inport  Port=4
  Down Sample1 : xbsIndex_r4/Down Sample  sample_ratio=4  sample_phase=First Value of Frame  en=off  latency=0  dbl_ovrd=off  block_type=dsamp
      settings: xl_area=[5 5 0 4 0 0 0]  block_version=VER_STRING_GOES_HERE
  Down Sample2 : xbsIndex_r4/Down Sample  sample_ratio=length(coef2)  sample_phase=First Value of Frame  en=off  latency=0  dbl_ovrd=off  block_type=dsamp
      settings: xl_area=[5 5 0 4 0 0 0]  block_version=VER_STRING_GOES_HERE
  MAC : Subsystem
  R : xbsIndex_r4/Addressable Shift Register  infer_latency=off  depth=length(coef2)  initVector=[0]  en=off  dbl_ovrd=off  use_behavioral_HDL=off  use_rpm=off  block_type=addrsr
      settings: infoedit="Delay of  configurable length.  Any element  in the delay     line   can    be   addressed   and   driven    onto   the   output     port.<P><P>Hardware  notes: Implemented  using SRL16s.     If Virtex-4, Virtex-II or Spartan-3 devices are used, multiple SRLC16s are cascaded together."  xl_area=[1 0 0 2 0 0 0]  block_version=VER_STRING_GOES_HERE
  ROM1 : xbsIndex_r4/ROM  depth=length(coef2)  initVector=coef2  distributed_mem=Distributed memory  rst=off  init_reg=0  en=off  latency=1  arith_type=Unsigned  n_bits=1  bin_pt=0  dbl_ovrd=off  optimize=Area  use_rpm=off  block_type=sprom
      settings: xl_area=[1 1 0 1 0 0 0]  block_version=VER_STRING_GOES_HERE
  yn : Outport
  xnz : Outport  Port=2
  wires:
  Down Sample1/1 -> R/1
  LD/1 -> MAC/3(ld)
  ROM1/1 -> MAC/2(coeff)
  Down Sample2/1 -> yn/1
  MAC/1(yn) -> Down Sample2/1
  R/1 -> MAC/1(a), xnz/1
  CAddr/1 -> ROM1/1
  DAddr/1 -> R/2
  xn/1 -> Down Sample1/1

== system ofdm_txrx_alamouti/OFDM Rx Alamouti/Long Corr 4x/C1/Cross C6 ==
  xn : Inport
  DAddr : Inport  Port=2
  CAddr : Inport  Port=3
  LD : Inport  Port=4
  Down Sample1 : xbsIndex_r4/Down Sample  sample_ratio=4  sample_phase=First Value of Frame  en=off  latency=0  dbl_ovrd=off  block_type=dsamp
      settings: xl_area=[5 5 0 4 0 0 0]  block_version=VER_STRING_GOES_HERE
  Down Sample2 : xbsIndex_r4/Down Sample  sample_ratio=length(coef2)  sample_phase=First Value of Frame  en=off  latency=0  dbl_ovrd=off  block_type=dsamp
      settings: xl_area=[5 5 0 4 0 0 0]  block_version=VER_STRING_GOES_HERE
  MAC : Subsystem
  R : xbsIndex_r4/Addressable Shift Register  infer_latency=off  depth=length(coef2)  initVector=[0]  en=off  dbl_ovrd=off  use_behavioral_HDL=off  use_rpm=off  block_type=addrsr
      settings: infoedit="Delay of  configurable length.  Any element  in the delay     line   can    be   addressed   and   driven    onto   the   output     port.<P><P>Hardware  notes: Implemented  using SRL16s.     If Virtex-4, Virtex-II or Spartan-3 devices are used, multiple SRLC16s are cascaded together."  xl_area=[1 0 0 2 0 0 0]  block_version=VER_STRING_GOES_HERE
  ROM1 : xbsIndex_r4/ROM  depth=length(coef2)  initVector=coef2  distributed_mem=Distributed memory  rst=off  init_reg=0  en=off  latency=1  arith_type=Unsigned  n_bits=1  bin_pt=0  dbl_ovrd=off  optimize=Area  use_rpm=off  block_type=sprom
      settings: xl_area=[1 1 0 1 0 0 0]  block_version=VER_STRING_GOES_HERE
  yn : Outport
  xnz : Outport  Port=2
  wires:
  Down Sample1/1 -> R/1
  LD/1 -> MAC/3(ld)
  ROM1/1 -> MAC/2(coeff)
  Down Sample2/1 -> yn/1
  MAC/1(yn) -> Down Sample2/1
  R/1 -> MAC/1(a), xnz/1
  CAddr/1 -> ROM1/1
  DAddr/1 -> R/2
  xn/1 -> Down Sample1/1

== system ofdm_txrx_alamouti/OFDM Rx Alamouti/Long Corr 4x/C1/Cross C7 ==
  xn : Inport
  DAddr : Inport  Port=2
  CAddr : Inport  Port=3
  LD : Inport  Port=4
  Down Sample1 : xbsIndex_r4/Down Sample  sample_ratio=4  sample_phase=First Value of Frame  en=off  latency=0  dbl_ovrd=off  block_type=dsamp
      settings: xl_area=[5 5 0 4 0 0 0]  block_version=VER_STRING_GOES_HERE
  Down Sample2 : xbsIndex_r4/Down Sample  sample_ratio=length(coef2)  sample_phase=First Value of Frame  en=off  latency=0  dbl_ovrd=off  block_type=dsamp
      settings: xl_area=[5 5 0 4 0 0 0]  block_version=VER_STRING_GOES_HERE
  MAC : Subsystem
  R : xbsIndex_r4/Addressable Shift Register  infer_latency=off  depth=length(coef2)  initVector=[0]  en=off  dbl_ovrd=off  use_behavioral_HDL=off  use_rpm=off  block_type=addrsr
      settings: infoedit="Delay of  configurable length.  Any element  in the delay     line   can    be   addressed   and   driven    onto   the   output     port.<P><P>Hardware  notes: Implemented  using SRL16s.     If Virtex-4, Virtex-II or Spartan-3 devices are used, multiple SRLC16s are cascaded together."  xl_area=[1 0 0 2 0 0 0]  block_version=VER_STRING_GOES_HERE
  ROM1 : xbsIndex_r4/ROM  depth=length(coef2)  initVector=coef2  distributed_mem=Distributed memory  rst=off  init_reg=0  en=off  latency=1  arith_type=Unsigned  n_bits=1  bin_pt=0  dbl_ovrd=off  optimize=Area  use_rpm=off  block_type=sprom
      settings: xl_area=[1 1 0 1 0 0 0]  block_version=VER_STRING_GOES_HERE
  yn : Outport
  xnz : Outport  Port=2
  wires:
  Down Sample1/1 -> R/1
  LD/1 -> MAC/3(ld)
  ROM1/1 -> MAC/2(coeff)
  Down Sample2/1 -> yn/1
  MAC/1(yn) -> Down Sample2/1
  R/1 -> MAC/1(a), xnz/1
  CAddr/1 -> ROM1/1
  DAddr/1 -> R/2
  xn/1 -> Down Sample1/1

== system ofdm_txrx_alamouti/OFDM Rx Alamouti/Long Corr 4x/C1/Cross C8 ==
  xn : Inport
  DAddr : Inport  Port=2
  CAddr : Inport  Port=3
  LD : Inport  Port=4
  Down Sample1 : xbsIndex_r4/Down Sample  sample_ratio=4  sample_phase=First Value of Frame  en=off  latency=0  dbl_ovrd=off  block_type=dsamp
      settings: xl_area=[5 5 0 4 0 0 0]  block_version=VER_STRING_GOES_HERE
  Down Sample2 : xbsIndex_r4/Down Sample  sample_ratio=length(coef2)  sample_phase=First Value of Frame  en=off  latency=0  dbl_ovrd=off  block_type=dsamp
      settings: xl_area=[5 5 0 4 0 0 0]  block_version=VER_STRING_GOES_HERE
  MAC : Subsystem
  R : xbsIndex_r4/Addressable Shift Register  infer_latency=off  depth=length(coef2)  initVector=[0]  en=off  dbl_ovrd=off  use_behavioral_HDL=off  use_rpm=off  block_type=addrsr
      settings: infoedit="Delay of  configurable length.  Any element  in the delay     line   can    be   addressed   and   driven    onto   the   output     port.<P><P>Hardware  notes: Implemented  using SRL16s.     If Virtex-4, Virtex-II or Spartan-3 devices are used, multiple SRLC16s are cascaded together."  xl_area=[1 0 0 2 0 0 0]  block_version=VER_STRING_GOES_HERE
  ROM1 : xbsIndex_r4/ROM  depth=length(coef2)  initVector=coef2  distributed_mem=Distributed memory  rst=off  init_reg=0  en=off  latency=1  arith_type=Unsigned  n_bits=1  bin_pt=0  dbl_ovrd=off  optimize=Area  use_rpm=off  block_type=sprom
      settings: xl_area=[1 1 0 1 0 0 0]  block_version=VER_STRING_GOES_HERE
  yn : Outport
  xnz : Outport  Port=2
  wires:
  Down Sample1/1 -> R/1
  LD/1 -> MAC/3(ld)
  ROM1/1 -> MAC/2(coeff)
  Down Sample2/1 -> yn/1
  MAC/1(yn) -> Down Sample2/1
  R/1 -> MAC/1(a), xnz/1
  CAddr/1 -> ROM1/1
  DAddr/1 -> R/2
  xn/1 -> Down Sample1/1

== system ofdm_txrx_alamouti/OFDM Rx Alamouti/Long Corr 4x/C1/Cross C9 ==
  xn : Inport
  DAddr : Inport  Port=2
  CAddr : Inport  Port=3
  LD : Inport  Port=4
  Down Sample1 : xbsIndex_r4/Down Sample  sample_ratio=4  sample_phase=First Value of Frame  en=off  latency=0  dbl_ovrd=off  block_type=dsamp
      settings: xl_area=[5 5 0 4 0 0 0]  block_version=VER_STRING_GOES_HERE
  Down Sample2 : xbsIndex_r4/Down Sample  sample_ratio=length(coef2)  sample_phase=First Value of Frame  en=off  latency=0  dbl_ovrd=off  block_type=dsamp
      settings: xl_area=[5 5 0 4 0 0 0]  block_version=VER_STRING_GOES_HERE
  MAC : Subsystem
  R : xbsIndex_r4/Addressable Shift Register  infer_latency=off  depth=length(coef2)  initVector=[0]  en=off  dbl_ovrd=off  use_behavioral_HDL=off  use_rpm=off  block_type=addrsr
      settings: infoedit="Delay of  configurable length.  Any element  in the delay     line   can    be   addressed   and   driven    onto   the   output     port.<P><P>Hardware  notes: Implemented  using SRL16s.     If Virtex-4, Virtex-II or Spartan-3 devices are used, multiple SRLC16s are cascaded together."  xl_area=[1 0 0 2 0 0 0]  block_version=VER_STRING_GOES_HERE
  ROM1 : xbsIndex_r4/ROM  depth=length(coef2)  initVector=coef2  distributed_mem=Distributed memory  rst=off  init_reg=0  en=off  latency=1  arith_type=Unsigned  n_bits=1  bin_pt=0  dbl_ovrd=off  optimize=Area  use_rpm=off  block_type=sprom
      settings: xl_area=[1 1 0 1 0 0 0]  block_version=VER_STRING_GOES_HERE
  yn : Outport
  xnz : Outport  Port=2
  wires:
  Down Sample1/1 -> R/1
  LD/1 -> MAC/3(ld)
  ROM1/1 -> MAC/2(coeff)
  Down Sample2/1 -> yn/1
  MAC/1(yn) -> Down Sample2/1
  R/1 -> MAC/1(a), xnz/1
  CAddr/1 -> ROM1/1
  DAddr/1 -> R/2
  xn/1 -> Down Sample1/1
